simulink model "AcasXuClosedLoop_all_comp"  (Simulink 10.0)
settings: Solver=VariableStepAuto  StartTime=0.0  StopTime=20  RelTol=1e-3
libraries: neural x14040, simulink x2
(part 21 of 534)

== system AcasXuClosedLoop_all_comp/networks/Networks_m_5/AcasXu_1_5/Layer 3 ==
  "a{2} " : Inport  PortDimensions=50
      mask Display: e = 0.06;c1 = [120 230 180]/255;c2 = ([120 230 180]+0)/(256+128);c3 = ([120 230 180]+128)/(256+128);patch([0 1 1 0],[0 0 1 1],c1);patch([0 1 1 1-e 1-e 0+e],[0 0 1 1-e 0+e 0+e],c2);patch([0 0 1 1-e 0+e 0+e],[0 1 1 1-e 1-e 0+e],c3);plot([0 1 1 0 0],[0 0 1 1 0]);
  Delays 1 : Subsystem
      mask Display: e = 0.06;c1 = [120 150 230]/255;c2 = ([120 150 230]+0)/383;c3 = ([120 150 230]+128)/383;patch([0 1 1 0],[0 0 1 1],c1);patch([0 1 1 1-e 1-e 0+e],[0 0 1 1-e 0+e 0+e],c2);patch([0 0 1 1-e 0+e 0+e],[0 1 1 1-e 1-e 0+e],c3);plot([0 1 1 0 0],[0 0 1 1 0]);disp('0');
  "LW{3,2}" : Subsystem
      mask Display: e = 0.06;c1 = [120 150 230]/255;c2 = ([120 150 230]+0)/383;c3 = ([120 150 230]+128)/383;patch([0 1 1 0],[0 0 1 1],c1);patch([0 1 1 1-e 1-e 0+e],[0 0 1 1-e 0+e 0+e],c2);patch([0 0 1 1-e 0+e 0+e],[0 1 1 1-e 1-e 0+e],c3);plot([0 1 1 0 0],[0 0 1 1 0]);disp('W');
  "b{3}" : Constant  Value=[-0.417030999999999985039522698571090586483478546142578125;0.051416999999999997428279385758287389762699604034423828125;-0.1922000000000000097255536957163712941110134124755859375;-0.0247398999999999986976195742727213655598461627960205078125;0.321873000000000020204282691338448785245418548583984375;0.054871999999999997166266751946750446222722530364990234375;0.1382190000000000085211837586030014790594577789306640625;-0.00863861999999999963240071565451216883957386016845703125;0.2219170000000000031459279625778435729444026947021484375;0.27942699999999998095034925427171401679515838623046875;-0.12495699999999999862954069840270676650106906890869140625;-0.401002000000000025092816713367938064038753509521484375;-0.005846829999999999909199299708006947184912860393524169921875;-0.1967190000000000049684700798025005497038364410400390625;-0.11063299999999999523314642146942787803709506988525390625;-0.503530000000000033111291486420668661594390869140625;-0.278268000000000015337064951381762512028217315673828125;-0.326998999999999984122922569440561346709728240966796875;-0.4205360000000000209041672860621474683284759521484375;-0.49979099999999998527044908769312314689159393310546875;-0.0027478500000000000320909965267901498009450733661651611328125;-0.595558000000000031803892852622084319591522216796875;-0.2478019999999999944950701546986238099634647369384765625;-0.0493603999999999987213783469996997155249118804931640625;-0.294870000000000020978774273316957987844944000244140625;0.0196781000000000004523936780742587870918214321136474609375;-0.2268429999999999890913926492430618964135646820068359375;0.323292999999999997040589505559182725846767425537109375;0.1608799999999999952304818862103275023400783538818359375;-0.038599300000000003052491592825390398502349853515625;-0.21826000000000000955679979597334749996662139892578125;0.269465999999999983316456564352847635746002197265625;0.1498879999999999934612304741676780395209789276123046875;-0.332399999999999973265829567026230506598949432373046875;0.2423619999999999941042716500305687077343463897705078125;-0.0757377999999999940161643507963162846863269805908203125;0.1714729999999999865423205847037024796009063720703125;-0.08564599999999999990762944435118697583675384521484375;-0.12559500000000001218580791828571818768978118896484375;-0.018844699999999998951683011227942188270390033721923828125;-0.1395280000000000131255006863284506835043430328369140625;0.291113000000000010647482895365101285278797149658203125;-0.03876670000000000115836229497290332801640033721923828125;0.317319999999999990958343687452725134789943695068359375;0.040440799999999998970423575883614830672740936279296875;-0.407876999999999989565679925362928770482540130615234375;-0.04297800000000000231192842647942597977817058563232421875;0.10669399999999999717470444693390163592994213104248046875;0.1600880000000000080717654782347381114959716796875;0.076302499999999995328181512377341277897357940673828125]
      mask Display: e = 0.06;c1 = [120 150 230]/255;c2 = ([120 150 230]+0)/383;c3 = ([120 150 230]+128)/383;patch([0 1 1 0],[0 0 1 1],c1);patch([0 1 1 1-e 1-e 0+e],[0 0 1 1-e 0+e 0+e],c2);patch([0 0 1 1-e 0+e 0+e],[0 1 1 1-e 1-e 0+e],c3);plot([0 1 1 0 0],[0 0 1 1 0]);disp('b');
  netsum : Sum  Inputs=2  SaturateOnIntegerOverflow=on  InputSameDT=on  OutDataTypeStr=Inherit: Same as first input
      mask Display: e = 0.06;c1 = [120 150 230]/255;c2 = ([120 150 230]+0)/383;c3 = ([120 150 230]+128)/383;patch([0 1 1 0],[0 0 1 1],c1);patch([0 1 1 1-e 1-e 0+e],[0 0 1 1-e 0+e 0+e],c2);patch([0 0 1 1-e 0+e 0+e],[0 1 1 1-e 1-e 0+e],c3);plot([0 1 1 0 0],[0 0 1 1 0]);disp('+');
  poslin : neural/Transfer Functions/poslin
      settings: SourceProductName=Deep Learning Toolbox  SourceProductBaseCode=NN
  "a{3}" : Outport  InitialOutput=[0;0;0;0;0;0;0;0;0;0;0;0;0;0;0;0;0;0;0;0;0;0;0;0;0;0;0;0;0;0;0;0;0;0;0;0;0;0;0;0;0;0;0;0;0;0;0;0;0;0]
      mask Display: e = 0.06;c1 = [120 230 180]/255;c2 = ([120 230 180]+0)/(256+128);c3 = ([120 230 180]+128)/(256+128);patch([0 1 1 0],[0 0 1 1],c1);patch([0 1 1 1-e 1-e 0+e],[0 0 1 1-e 0+e 0+e],c2);patch([0 0 1 1-e 0+e 0+e],[0 1 1 1-e 1-e 0+e],c3);plot([0 1 1 0 0],[0 0 1 1 0]);
  wires:
  poslin/1 -> "a{3}"/1
  netsum/1 -> poslin/1
  "b{3}"/1 -> netsum/2
  "LW{3,2}"/1("lz{3,2}") -> netsum/1
  Delays 1/1("pd{3,2}") -> "LW{3,2}"/1("ad{3,2}")
  "a{2} "/1 -> Delays 1/1("p{3}")

== system AcasXuClosedLoop_all_comp/networks/Networks_m_5/AcasXu_1_5/Layer 4 ==
  "a{3} " : Inport  PortDimensions=50
      mask Display: e = 0.06;c1 = [120 230 180]/255;c2 = ([120 230 180]+0)/(256+128);c3 = ([120 230 180]+128)/(256+128);patch([0 1 1 0],[0 0 1 1],c1);patch([0 1 1 1-e 1-e 0+e],[0 0 1 1-e 0+e 0+e],c2);patch([0 0 1 1-e 0+e 0+e],[0 1 1 1-e 1-e 0+e],c3);plot([0 1 1 0 0],[0 0 1 1 0]);
  Delays 1 : Subsystem
      mask Display: e = 0.06;c1 = [120 150 230]/255;c2 = ([120 150 230]+0)/383;c3 = ([120 150 230]+128)/383;patch([0 1 1 0],[0 0 1 1],c1);patch([0 1 1 1-e 1-e 0+e],[0 0 1 1-e 0+e 0+e],c2);patch([0 0 1 1-e 0+e 0+e],[0 1 1 1-e 1-e 0+e],c3);plot([0 1 1 0 0],[0 0 1 1 0]);disp('0');
  "LW{4,3}" : Subsystem
      mask Display: e = 0.06;c1 = [120 150 230]/255;c2 = ([120 150 230]+0)/383;c3 = ([120 150 230]+128)/383;patch([0 1 1 0],[0 0 1 1],c1);patch([0 1 1 1-e 1-e 0+e],[0 0 1 1-e 0+e 0+e],c2);patch([0 0 1 1-e 0+e 0+e],[0 1 1 1-e 1-e 0+e],c3);plot([0 1 1 0 0],[0 0 1 1 0]);disp('W');
  "b{4}" : Constant  Value=[-0.3807139999999999968594011079403571784496307373046875;0.769236999999999948585127640399150550365447998046875;0.11294200000000000072564176889500231482088565826416015625;-0.298995999999999984009235731718945316970348358154296875;-0.1376940000000000108304476498233270831406116485595703125;-0.2200870000000000048512305284020840190351009368896484375;-0.0667924000000000017696066834105295129120349884033203125;-0.1035719999999999973994135871180333197116851806640625;-0.4381939999999999724167309977929107844829559326171875;-0.197091999999999989423571378210908733308315277099609375;-0.023435299999999999187583199500295449979603290557861328125;-0.0131678999999999997438937526794688892550766468048095703125;0.295812000000000019372947690499131567776203155517578125;0.2067740000000000133439925775746814906597137451171875;-0.825668999999999986272314345114864408969879150390625;-0.1489729999999999943138817570797982625663280487060546875;-0.12124100000000000154631862869791802950203418731689453125;-0.164737999999999995548449760462972335517406463623046875;-0.52807199999999998585309413101640529930591583251953125;0.2460940000000000071889161290528136305510997772216796875;0.11102299999999999668975902977763325907289981842041015625;0.08866369999999999806927775125586776994168758392333984375;0.4474650000000000016342482922482304275035858154296875;0.0499510999999999982801313080926775000989437103271484375;0.477864999999999984225951266125775873661041259765625;-0.1715270000000000127915456005212035961449146270751953125;0.338029999999999997140065488565596751868724822998046875;-0.318321000000000020602186623364104889333248138427734375;-0.302983999999999975560882603531354106962680816650390625;0.07483410000000000061870508716310723684728145599365234375;-0.0716896999999999950947682236801483668386936187744140625;-0.68828999999999995740296299118199385702610015869140625;0.436628999999999989345411677277297712862491607666015625;-0.98602800000000001556799134050379507243633270263671875;-0.00702441000000000002889688488494357443414628505706787109375;-0.67567399999999999682387397115235216915607452392578125;-0.472779000000000004799716180059476755559444427490234375;0.76656299999999999439381781485280953347682952880859375;-0.01495920000000000062156946256664014072157442569732666015625;-0.01252909999999999961228791534040283295325934886932373046875;-0.339034000000000002028599510595086030662059783935546875;0.1361610000000000042508219166847993619740009307861328125;0.30265799999999998259880840123514644801616668701171875;-0.329135000000000010889067425523535348474979400634765625;-0.01919679999999999997495336856445646844804286956787109375;0.10113600000000000367350594387971796095371246337890625;-0.0011569399999999999649469284435099325492046773433685302734375;0.313553000000000026137314534935285337269306182861328125;-1.142490000000000005542233338928781449794769287109375;0.267479999999999995541344333105371333658695220947265625]
      mask Display: e = 0.06;c1 = [120 150 230]/255;c2 = ([120 150 230]+0)/383;c3 = ([120 150 230]+128)/383;patch([0 1 1 0],[0 0 1 1],c1);patch([0 1 1 1-e 1-e 0+e],[0 0 1 1-e 0+e 0+e],c2);patch([0 0 1 1-e 0+e 0+e],[0 1 1 1-e 1-e 0+e],c3);plot([0 1 1 0 0],[0 0 1 1 0]);disp('b');
  netsum : Sum  Inputs=2  SaturateOnIntegerOverflow=on  InputSameDT=on  OutDataTypeStr=Inherit: Same as first input
      mask Display: e = 0.06;c1 = [120 150 230]/255;c2 = ([120 150 230]+0)/383;c3 = ([120 150 230]+128)/383;patch([0 1 1 0],[0 0 1 1],c1);patch([0 1 1 1-e 1-e 0+e],[0 0 1 1-e 0+e 0+e],c2);patch([0 0 1 1-e 0+e 0+e],[0 1 1 1-e 1-e 0+e],c3);plot([0 1 1 0 0],[0 0 1 1 0]);disp('+');
  poslin : neural/Transfer Functions/poslin
      settings: SourceProductName=Deep Learning Toolbox  SourceProductBaseCode=NN
  "a{4}" : Outport  InitialOutput=[0;0;0;0;0;0;0;0;0;0;0;0;0;0;0;0;0;0;0;0;0;0;0;0;0;0;0;0;0;0;0;0;0;0;0;0;0;0;0;0;0;0;0;0;0;0;0;0;0;0]
      mask Display: e = 0.06;c1 = [120 230 180]/255;c2 = ([120 230 180]+0)/(256+128);c3 = ([120 230 180]+128)/(256+128);patch([0 1 1 0],[0 0 1 1],c1);patch([0 1 1 1-e 1-e 0+e],[0 0 1 1-e 0+e 0+e],c2);patch([0 0 1 1-e 0+e 0+e],[0 1 1 1-e 1-e 0+e],c3);plot([0 1 1 0 0],[0 0 1 1 0]);
  wires:
  poslin/1 -> "a{4}"/1
  netsum/1 -> poslin/1
  "b{4}"/1 -> netsum/2
  "LW{4,3}"/1("lz{4,3}") -> netsum/1
  Delays 1/1("pd{4,3}") -> "LW{4,3}"/1("ad{4,3}")
  "a{3} "/1 -> Delays 1/1("p{4}")

== system AcasXuClosedLoop_all_comp/networks/Networks_m_5/AcasXu_1_5/Layer 5 ==
  "a{4} " : Inport  PortDimensions=50
      mask Display: e = 0.06;c1 = [120 230 180]/255;c2 = ([120 230 180]+0)/(256+128);c3 = ([120 230 180]+128)/(256+128);patch([0 1 1 0],[0 0 1 1],c1);patch([0 1 1 1-e 1-e 0+e],[0 0 1 1-e 0+e 0+e],c2);patch([0 0 1 1-e 0+e 0+e],[0 1 1 1-e 1-e 0+e],c3);plot([0 1 1 0 0],[0 0 1 1 0]);
  Delays 1 : Subsystem
      mask Display: e = 0.06;c1 = [120 150 230]/255;c2 = ([120 150 230]+0)/383;c3 = ([120 150 230]+128)/383;patch([0 1 1 0],[0 0 1 1],c1);patch([0 1 1 1-e 1-e 0+e],[0 0 1 1-e 0+e 0+e],c2);patch([0 0 1 1-e 0+e 0+e],[0 1 1 1-e 1-e 0+e],c3);plot([0 1 1 0 0],[0 0 1 1 0]);disp('0');
  "LW{5,4}" : Subsystem
      mask Display: e = 0.06;c1 = [120 150 230]/255;c2 = ([120 150 230]+0)/383;c3 = ([120 150 230]+128)/383;patch([0 1 1 0],[0 0 1 1],c1);patch([0 1 1 1-e 1-e 0+e],[0 0 1 1-e 0+e 0+e],c2);patch([0 0 1 1-e 0+e 0+e],[0 1 1 1-e 1-e 0+e],c3);plot([0 1 1 0 0],[0 0 1 1 0]);disp('W');
  "b{5}" : Constant  Value=[-0.055169999999999996820321257473551668226718902587890625;-0.41405999999999998362198994072969071567058563232421875;0.0014745900000000000153177470707532847882248461246490478515625;0.270907999999999982154719191385083831846714019775390625;0.266349000000000002419398015263141132891178131103515625;0.339114999999999999769073610877967439591884613037109375;-1.0929800000000000625988150204648263752460479736328125;-0.11807499999999999940047956670241546817123889923095703125;0.32457999999999997964295062047312967479228973388671875;0.35002699999999997704236420759116299450397491455078125;-0.435273999999999994248156554021988995373249053955078125;-0.3247189999999999798774297232739627361297607421875;0.1182249999999999967581487680945429019629955291748046875;-0.124670000000000003037570195374428294599056243896484375;-0.011235900000000000054090065759737626649439334869384765625;0.400903000000000009350742402602918446063995361328125;-0.07515719999999999345607903933341731317341327667236328125;0.1054920000000000024353852268177433870732784271240234375;0.1665290000000000103508313031852594576776027679443359375;-1.8045299999999999673860884286114014685153961181640625;0.2429929999999999867821287580227362923324108123779296875;0.9974089999999999900381908446433953940868377685546875;0.1574979999999999991100452234604745171964168548583984375;-0.30226399999999997714184019059757702052593231201171875;-0.313302000000000024915181029427913017570972442626953125;0.2015280000000000126814114764783880673348903656005859375;1.3892700000000000049027448767446912825107574462890625;-0.1532509999999999983355536414819653145968914031982421875;-0.150237000000000009425349389857728965580463409423828125;-0.2088440000000000018598456108520622365176677703857421875;-0.02673419999999999963069541308868792839348316192626953125;0.71494599999999997042010591030702926218509674072265625;0.0299004999999999999948929740867242799140512943267822265625;0.08596589999999999787139159934667986817657947540283203125;0.179721999999999992869703646647394634783267974853515625;0.05434239999999999903845804283264442346990108489990234375;0.6289379999999999970583530739531852304935455322265625;0.355820000000000025153212845907546579837799072265625;0.042970500000000001750155576019096770323812961578369140625;-0.1282999999999999973798736618846305646002292633056640625;-0.49241699999999999359800995080149732530117034912109375;0.7115080000000000293169932774617336690425872802734375;-0.09608129999999999448778709165708278305828571319580078125;0.354057000000000010597744903861894272267818450927734375;0.2978899999999999881339363128063268959522247314453125;-0.055887399999999996802468871237579151056706905364990234375;0.64739500000000005375255796025157906115055084228515625;0.09175229999999999497273250881335115991532802581787109375;-0.1348080000000000111537445945941726677119731903076171875;-0.457637000000000015997869695638655684888362884521484375]
      mask Display: e = 0.06;c1 = [120 150 230]/255;c2 = ([120 150 230]+0)/383;c3 = ([120 150 230]+128)/383;patch([0 1 1 0],[0 0 1 1],c1);patch([0 1 1 1-e 1-e 0+e],[0 0 1 1-e 0+e 0+e],c2);patch([0 0 1 1-e 0+e 0+e],[0 1 1 1-e 1-e 0+e],c3);plot([0 1 1 0 0],[0 0 1 1 0]);disp('b');
  netsum : Sum  Inputs=2  SaturateOnIntegerOverflow=on  InputSameDT=on  OutDataTypeStr=Inherit: Same as first input
      mask Display: e = 0.06;c1 = [120 150 230]/255;c2 = ([120 150 230]+0)/383;c3 = ([120 150 230]+128)/383;patch([0 1 1 0],[0 0 1 1],c1);patch([0 1 1 1-e 1-e 0+e],[0 0 1 1-e 0+e 0+e],c2);patch([0 0 1 1-e 0+e 0+e],[0 1 1 1-e 1-e 0+e],c3);plot([0 1 1 0 0],[0 0 1 1 0]);disp('+');
  poslin : neural/Transfer Functions/poslin
      settings: SourceProductName=Deep Learning Toolbox  SourceProductBaseCode=NN
  "a{5}" : Outport  InitialOutput=[0;0;0;0;0;0;0;0;0;0;0;0;0;0;0;0;0;0;0;0;0;0;0;0;0;0;0;0;0;0;0;0;0;0;0;0;0;0;0;0;0;0;0;0;0;0;0;0;0;0]
      mask Display: e = 0.06;c1 = [120 230 180]/255;c2 = ([120 230 180]+0)/(256+128);c3 = ([120 230 180]+128)/(256+128);patch([0 1 1 0],[0 0 1 1],c1);patch([0 1 1 1-e 1-e 0+e],[0 0 1 1-e 0+e 0+e],c2);patch([0 0 1 1-e 0+e 0+e],[0 1 1 1-e 1-e 0+e],c3);plot([0 1 1 0 0],[0 0 1 1 0]);
  wires:
  poslin/1 -> "a{5}"/1
  netsum/1 -> poslin/1
  "b{5}"/1 -> netsum/2
  "LW{5,4}"/1("lz{5,4}") -> netsum/1
  Delays 1/1("pd{5,4}") -> "LW{5,4}"/1("ad{5,4}")
  "a{4} "/1 -> Delays 1/1("p{5}")

== system AcasXuClosedLoop_all_comp/networks/Networks_m_5/AcasXu_1_5/Layer 6 ==
  "a{5} " : Inport  PortDimensions=50
      mask Display: e = 0.06;c1 = [120 230 180]/255;c2 = ([120 230 180]+0)/(256+128);c3 = ([120 230 180]+128)/(256+128);patch([0 1 1 0],[0 0 1 1],c1);patch([0 1 1 1-e 1-e 0+e],[0 0 1 1-e 0+e 0+e],c2);patch([0 0 1 1-e 0+e 0+e],[0 1 1 1-e 1-e 0+e],c3);plot([0 1 1 0 0],[0 0 1 1 0]);
  Delays 1 : Subsystem
      mask Display: e = 0.06;c1 = [120 150 230]/255;c2 = ([120 150 230]+0)/383;c3 = ([120 150 230]+128)/383;patch([0 1 1 0],[0 0 1 1],c1);patch([0 1 1 1-e 1-e 0+e],[0 0 1 1-e 0+e 0+e],c2);patch([0 0 1 1-e 0+e 0+e],[0 1 1 1-e 1-e 0+e],c3);plot([0 1 1 0 0],[0 0 1 1 0]);disp('0');
  "LW{6,5}" : Subsystem
      mask Display: e = 0.06;c1 = [120 150 230]/255;c2 = ([120 150 230]+0)/383;c3 = ([120 150 230]+128)/383;patch([0 1 1 0],[0 0 1 1],c1);patch([0 1 1 1-e 1-e 0+e],[0 0 1 1-e 0+e 0+e],c2);patch([0 0 1 1-e 0+e 0+e],[0 1 1 1-e 1-e 0+e],c3);plot([0 1 1 0 0],[0 0 1 1 0]);disp('W');
  "b{6}" : Constant  Value=[-0.212903000000000008906653192752855829894542694091796875;0.1829119999999999912621007069901679642498493194580078125;0.29444700000000001427480356142041273415088653564453125;0.42106100000000001859490339484182186424732208251953125;-0.05925729999999999886295398709989967755973339080810546875;-0.96771399999999996310862115933559834957122802734375;-0.017947999999999998677058243856663466431200504302978515625;0.51803999999999994496846511538024060428142547607421875;0.0897030000000000049542592250872985459864139556884765625;0.061536000000000000420552481728009297512471675872802734375;0.311950000000000005062616992290713824331760406494140625;-0.413208999999999992969179629653808660805225372314453125;0.3383249999999999868549593884381465613842010498046875;-0.486316000000000026037838551928871311247348785400390625;0.2037269999999999914308546067331917583942413330078125;-0.0403260000000000007336353746723034419119358062744140625;-0.307506000000000001559641304993419907987117767333984375;0.06562410000000000465103511260167579166591167449951171875;-0.034883900000000002405986521125669241882860660552978515625;0.34183099999999999596411726088263094425201416015625;-0.0035042400000000000458155735572063349536620080471038818359375;0.0245180000000000015980550216454503242857754230499267578125;0.661869999999999958362195684458129107952117919921875;-0.623188999999999992951416061259806156158447265625;-0.11044900000000000550581802372107631526887416839599609375;-0.057677899999999997227018155854239012114703655242919921875;-0.03260649999999999659738847412882023490965366363525390625;-0.1454660000000000119602105996818863786756992340087890625;-0.32797799999999999176480969254043884575366973876953125;0.204077000000000008395062423005583696067333221435546875;-0.021273699999999999554756158204327221028506755828857421875;0.382120000000000015205614545266143977642059326171875;-0.1458250000000000101696429055664339102804660797119140625;-0.0122827999999999999680699858117804978974163532257080078125;-0.51167799999999996618527120517683215439319610595703125;-0.2363280000000000102833297432880499400198459625244140625;-0.0236589000000000000689670542897147242911159992218017578125;-0.57806900000000005501732402990455739200115203857421875;-0.05386180000000000134274813490264932624995708465576171875;1.28347999999999995424104781704954802989959716796875;-0.238109999999999988329335565140354447066783905029296875;-0.02524300000000000154809498553731827996671199798583984375;-0.16046199999999999352695567722548730671405792236328125;-0.01005679999999999942039696776419077650643885135650634765625;-0.32135999999999997900346215828903950750827789306640625;-0.94411999999999995925037410415825434029102325439453125;-0.569733999999999962682295517879538238048553466796875;-0.0232010999999999988074872447896268568001687526702880859375;0.5037599999999999855759824640699662268161773681640625;0.430217000000000016068923969214665703475475311279296875]
      mask Display: e = 0.06;c1 = [120 150 230]/255;c2 = ([120 150 230]+0)/383;c3 = ([120 150 230]+128)/383;patch([0 1 1 0],[0 0 1 1],c1);patch([0 1 1 1-e 1-e 0+e],[0 0 1 1-e 0+e 0+e],c2);patch([0 0 1 1-e 0+e 0+e],[0 1 1 1-e 1-e 0+e],c3);plot([0 1 1 0 0],[0 0 1 1 0]);disp('b');
  netsum : Sum  Inputs=2  SaturateOnIntegerOverflow=on  InputSameDT=on  OutDataTypeStr=Inherit: Same as first input
      mask Display: e = 0.06;c1 = [120 150 230]/255;c2 = ([120 150 230]+0)/383;c3 = ([120 150 230]+128)/383;patch([0 1 1 0],[0 0 1 1],c1);patch([0 1 1 1-e 1-e 0+e],[0 0 1 1-e 0+e 0+e],c2);patch([0 0 1 1-e 0+e 0+e],[0 1 1 1-e 1-e 0+e],c3);plot([0 1 1 0 0],[0 0 1 1 0]);disp('+');
  poslin : neural/Transfer Functions/poslin
      settings: SourceProductName=Deep Learning Toolbox  SourceProductBaseCode=NN
  "a{6}" : Outport  InitialOutput=[0;0;0;0;0;0;0;0;0;0;0;0;0;0;0;0;0;0;0;0;0;0;0;0;0;0;0;0;0;0;0;0;0;0;0;0;0;0;0;0;0;0;0;0;0;0;0;0;0;0]
      mask Display: e = 0.06;c1 = [120 230 180]/255;c2 = ([120 230 180]+0)/(256+128);c3 = ([120 230 180]+128)/(256+128);patch([0 1 1 0],[0 0 1 1],c1);patch([0 1 1 1-e 1-e 0+e],[0 0 1 1-e 0+e 0+e],c2);patch([0 0 1 1-e 0+e 0+e],[0 1 1 1-e 1-e 0+e],c3);plot([0 1 1 0 0],[0 0 1 1 0]);
  wires:
  poslin/1 -> "a{6}"/1
  netsum/1 -> poslin/1
  "b{6}"/1 -> netsum/2
  "LW{6,5}"/1("lz{6,5}") -> netsum/1
  Delays 1/1("pd{6,5}") -> "LW{6,5}"/1("ad{6,5}")
  "a{5} "/1 -> Delays 1/1("p{6}")

== system AcasXuClosedLoop_all_comp/networks/Networks_m_5/AcasXu_1_5/Layer 7 ==
  "a{6} " : Inport  PortDimensions=50
      mask Display: e = 0.06;c1 = [120 230 180]/255;c2 = ([120 230 180]+0)/(256+128);c3 = ([120 230 180]+128)/(256+128);patch([0 1 1 0],[0 0 1 1],c1);patch([0 1 1 1-e 1-e 0+e],[0 0 1 1-e 0+e 0+e],c2);patch([0 0 1 1-e 0+e 0+e],[0 1 1 1-e 1-e 0+e],c3);plot([0 1 1 0 0],[0 0 1 1 0]);
  Delays 1 : Subsystem
      mask Display: e = 0.06;c1 = [120 150 230]/255;c2 = ([120 150 230]+0)/383;c3 = ([120 150 230]+128)/383;patch([0 1 1 0],[0 0 1 1],c1);patch([0 1 1 1-e 1-e 0+e],[0 0 1 1-e 0+e 0+e],c2);patch([0 0 1 1-e 0+e 0+e],[0 1 1 1-e 1-e 0+e],c3);plot([0 1 1 0 0],[0 0 1 1 0]);disp('0');
  "LW{7,6}" : Subsystem
      mask Display: e = 0.06;c1 = [120 150 230]/255;c2 = ([120 150 230]+0)/383;c3 = ([120 150 230]+128)/383;patch([0 1 1 0],[0 0 1 1],c1);patch([0 1 1 1-e 1-e 0+e],[0 0 1 1-e 0+e 0+e],c2);patch([0 0 1 1-e 0+e 0+e],[0 1 1 1-e 1-e 0+e],c3);plot([0 1 1 0 0],[0 0 1 1 0]);disp('W');
  "b{7}" : Constant  Value=[-0.01177249999999999997835065101980944746173918247222900390625;-0.0173447000000000010888623336313685285858809947967529296875;-0.016608299999999999452260368570932769216597080230712890625;-0.016006499999999999894750857265535159967839717864990234375;-0.0142433999999999998109512233668283442966639995574951171875]
      mask Display: e = 0.06;c1 = [120 150 230]/255;c2 = ([120 150 230]+0)/383;c3 = ([120 150 230]+128)/383;patch([0 1 1 0],[0 0 1 1],c1);patch([0 1 1 1-e 1-e 0+e],[0 0 1 1-e 0+e 0+e],c2);patch([0 0 1 1-e 0+e 0+e],[0 1 1 1-e 1-e 0+e],c3);plot([0 1 1 0 0],[0 0 1 1 0]);disp('b');
  netsum : Sum  Inputs=2  SaturateOnIntegerOverflow=on  InputSameDT=on  OutDataTypeStr=Inherit: Same as first input
      mask Display: e = 0.06;c1 = [120 150 230]/255;c2 = ([120 150 230]+0)/383;c3 = ([120 150 230]+128)/383;patch([0 1 1 0],[0 0 1 1],c1);patch([0 1 1 1-e 1-e 0+e],[0 0 1 1-e 0+e 0+e],c2);patch([0 0 1 1-e 0+e 0+e],[0 1 1 1-e 1-e 0+e],c3);plot([0 1 1 0 0],[0 0 1 1 0]);disp('+');
  purelin : neural/Transfer Functions/purelin
      settings: SourceProductName=Deep Learning Toolbox
  "a{7}" : Outport  InitialOutput=[0;0;0;0;0]
      mask Display: e = 0.06;c1 = [120 230 180]/255;c2 = ([120 230 180]+0)/(256+128);c3 = ([120 230 180]+128)/(256+128);patch([0 1 1 0],[0 0 1 1],c1);patch([0 1 1 1-e 1-e 0+e],[0 0 1 1-e 0+e 0+e],c2);patch([0 0 1 1-e 0+e 0+e],[0 1 1 1-e 1-e 0+e],c3);plot([0 1 1 0 0],[0 0 1 1 0]);
  wires:
  purelin/1 -> "a{7}"/1
  netsum/1 -> purelin/1
  "b{7}"/1 -> netsum/2
  "LW{7,6}"/1("lz{7,6}") -> netsum/1
  Delays 1/1("pd{7,6}") -> "LW{7,6}"/1("ad{7,6}")
  "a{6} "/1 -> Delays 1/1("p{7}")

== system AcasXuClosedLoop_all_comp/networks/Networks_m_5/AcasXu_1_5/Process Input 1 ==
  x : Inport  PortDimensions=5
      mask Display: e = 0.06;c1 = [120 230 180]/255;c2 = ([120 230 180]+0)/(256+128);c3 = ([120 230 180]+128)/(256+128);patch([0 1 1 0],[0 0 1 1],c1);patch([0 1 1 1-e 1-e 0+e],[0 0 1 1-e 0+e 0+e],c2);patch([0 0 1 1-e 0+e 0+e],[0 1 1 1-e 1-e 0+e],c3);plot([0 1 1 0 0],[0 0 1 1 0]);
  p : Outport  PortDimensions=5
      mask Display: e = 0.06;c1 = [120 230 180]/255;c2 = ([120 230 180]+0)/(256+128);c3 = ([120 230 180]+128)/(256+128);patch([0 1 1 0],[0 0 1 1],c1);patch([0 1 1 1-e 1-e 0+e],[0 0 1 1-e 0+e 0+e],c2);patch([0 0 1 1-e 0+e 0+e],[0 1 1 1-e 1-e 0+e],c3);plot([0 1 1 0 0],[0 0 1 1 0]);
  wires:
  x/1 -> p/1

== system AcasXuClosedLoop_all_comp/networks/Networks_m_5/AcasXu_1_5/Process Output 1 ==
  a : Inport  PortDimensions=5
      mask Display: e = 0.06;c1 = [120 230 180]/255;c2 = ([120 230 180]+0)/(256+128);c3 = ([120 230 180]+128)/(256+128);patch([0 1 1 0],[0 0 1 1],c1);patch([0 1 1 1-e 1-e 0+e],[0 0 1 1-e 0+e 0+e],c2);patch([0 0 1 1-e 0+e 0+e],[0 1 1 1-e 1-e 0+e],c3);plot([0 1 1 0 0],[0 0 1 1 0]);
  y : Outport  PortDimensions=5
      mask Display: e = 0.06;c1 = [120 230 180]/255;c2 = ([120 230 180]+0)/(256+128);c3 = ([120 230 180]+128)/(256+128);patch([0 1 1 0],[0 0 1 1],c1);patch([0 1 1 1-e 1-e 0+e],[0 0 1 1-e 0+e 0+e],c2);patch([0 0 1 1-e 0+e 0+e],[0 1 1 1-e 1-e 0+e],c3);plot([0 1 1 0 0],[0 0 1 1 0]);
  wires:
  a/1 -> y/1

== system AcasXuClosedLoop_all_comp/networks/Networks_m_5/AcasXu_2_5/Layer 1 ==
  "p{1}" : Inport  PortDimensions=5
      mask Display: e = 0.06;c1 = [120 230 180]/255;c2 = ([120 230 180]+0)/(256+128);c3 = ([120 230 180]+128)/(256+128);patch([0 1 1 0],[0 0 1 1],c1);patch([0 1 1 1-e 1-e 0+e],[0 0 1 1-e 0+e 0+e],c2);patch([0 0 1 1-e 0+e 0+e],[0 1 1 1-e 1-e 0+e],c3);plot([0 1 1 0 0],[0 0 1 1 0]);
  Delays 1 : Subsystem
      mask Display: e = 0.06;c1 = [120 150 230]/255;c2 = ([120 150 230]+0)/383;c3 = ([120 150 230]+128)/383;patch([0 1 1 0],[0 0 1 1],c1);patch([0 1 1 1-e 1-e 0+e],[0 0 1 1-e 0+e 0+e],c2);patch([0 0 1 1-e 0+e 0+e],[0 1 1 1-e 1-e 0+e],c3);plot([0 1 1 0 0],[0 0 1 1 0]);disp('0');
  "IW{1,1}" : Subsystem
      mask Display: e = 0.06;c1 = [120 150 230]/255;c2 = ([120 150 230]+0)/383;c3 = ([120 150 230]+128)/383;patch([0 1 1 0],[0 0 1 1],c1);patch([0 1 1 1-e 1-e 0+e],[0 0 1 1-e 0+e 0+e],c2);patch([0 0 1 1-e 0+e 0+e],[0 1 1 1-e 1-e 0+e],c3);plot([0 1 1 0 0],[0 0 1 1 0]);disp('W');
  "b{1}" : Constant  Value=[-0.0934323999999999987853271932181087322533130645751953125;-0.68851399999999995937827179659507237374782562255859375;0.181541000000000007918998790046316571533679962158203125;-0.17247000000000001218580791828571818768978118896484375;-0.1560479999999999922710713917695102281868457794189453125;0.12035099999999999964561681053965003229677677154541015625;0.06389630000000000309423597855129628442227840423583984375;-0.086717199999999994286525861753034405410289764404296875;0.226731999999999989103827147118863649666309356689453125;-0.271637999999999990574650610142271034419536590576171875;0.1380530000000000090398799557078746147453784942626953125;-0.0289674000000000007870593066172659746371209621429443359375;-0.052192099999999998216626551084118545986711978912353515625;0.0257320000000000013995471448424723348580300807952880859375;-0.073908899999999999597122268824023194611072540283203125;-0.254815999999999986957988085123361088335514068603515625;-0.29719899999999999096189640113152563571929931640625;-0.37860199999999999409538986583356745541095733642578125;0.275386999999999992905230783435399644076824188232421875;0.07508850000000000246558329308754764497280120849609375;0.2205519999999999980477838334991247393190860748291015625;0.08967540000000000233182362308070878498256206512451171875;0.11587699999999999389554972140103927813470363616943359375;-0.68539600000000000523669996255193836987018585205078125;0.007177379999999999697635200135437116841785609722137451171875;0.119696999999999997843502796968095935881137847900390625;0.0220079999999999997850608224325696937739849090576171875;0.48674600000000001198685595227289013564586639404296875;0.0803882999999999958706808911301777698099613189697265625;-0.00837524000000000080901063626015456975437700748443603515625;0.051856699999999998629274244876796728931367397308349609375;0.007693120000000000340001360399355689878575503826141357421875;-0.1534590000000000120650156532065011560916900634765625;-0.143386999999999986687981845534523017704486846923828125;-0.07607619999999999660378335875066113658249378204345703125;-0.055888000000000000178079773149875109083950519561767578125;-0.000299668000000000004680311693761041169636882841587066650390625;0.1378749999999999975575093458246556110680103302001953125;-0.06373510000000000286224377532562357373535633087158203125;0.09505560000000000397957222730838111601769924163818359375;0.2410200000000000120081722343456931412220001220703125;-0.1569319999999999881712398064337321557104587554931640625;-0.258384000000000002561506562415161170065402984619140625;0.503395999999999954610530039644800126552581787109375;-0.278918999999999972505548839762923307716846466064453125;0.02316100000000000103117514527184539474546909332275390625;-0.54474699999999998123456634857575409114360809326171875;0.09261429999999999662652072629498434253036975860595703125;0.17898300000000000320454773827805183827877044677734375;-0.44224099999999999521804738833452574908733367919921875]
      mask Display: e = 0.06;c1 = [120 150 230]/255;c2 = ([120 150 230]+0)/383;c3 = ([120 150 230]+128)/383;patch([0 1 1 0],[0 0 1 1],c1);patch([0 1 1 1-e 1-e 0+e],[0 0 1 1-e 0+e 0+e],c2);patch([0 0 1 1-e 0+e 0+e],[0 1 1 1-e 1-e 0+e],c3);plot([0 1 1 0 0],[0 0 1 1 0]);disp('b');
  netsum : Sum  Inputs=2  SaturateOnIntegerOverflow=on  InputSameDT=on  OutDataTypeStr=Inherit: Same as first input
      mask Display: e = 0.06;c1 = [120 150 230]/255;c2 = ([120 150 230]+0)/383;c3 = ([120 150 230]+128)/383;patch([0 1 1 0],[0 0 1 1],c1);patch([0 1 1 1-e 1-e 0+e],[0 0 1 1-e 0+e 0+e],c2);patch([0 0 1 1-e 0+e 0+e],[0 1 1 1-e 1-e 0+e],c3);plot([0 1 1 0 0],[0 0 1 1 0]);disp('+');
  poslin : neural/Transfer Functions/poslin
      settings: SourceProductName=Deep Learning Toolbox  SourceProductBaseCode=NN
  "a{1}" : Outport  InitialOutput=[0;0;0;0;0;0;0;0;0;0;0;0;0;0;0;0;0;0;0;0;0;0;0;0;0;0;0;0;0;0;0;0;0;0;0;0;0;0;0;0;0;0;0;0;0;0;0;0;0;0]
      mask Display: e = 0.06;c1 = [120 230 180]/255;c2 = ([120 230 180]+0)/(256+128);c3 = ([120 230 180]+128)/(256+128);patch([0 1 1 0],[0 0 1 1],c1);patch([0 1 1 1-e 1-e 0+e],[0 0 1 1-e 0+e 0+e],c2);patch([0 0 1 1-e 0+e 0+e],[0 1 1 1-e 1-e 0+e],c3);plot([0 1 1 0 0],[0 0 1 1 0]);
  wires:
  poslin/1 -> "a{1}"/1
  netsum/1 -> poslin/1
  "b{1}"/1 -> netsum/2
  "IW{1,1}"/1("iz{1,1}") -> netsum/1
  Delays 1/1("pd{1,1}") -> "IW{1,1}"/1("pd{1,1}")
  "p{1}"/1 -> Delays 1/1("p{1}")

== system AcasXuClosedLoop_all_comp/networks/Networks_m_5/AcasXu_2_5/Layer 2 ==
  "a{1} " : Inport  PortDimensions=50
      mask Display: e = 0.06;c1 = [120 230 180]/255;c2 = ([120 230 180]+0)/(256+128);c3 = ([120 230 180]+128)/(256+128);patch([0 1 1 0],[0 0 1 1],c1);patch([0 1 1 1-e 1-e 0+e],[0 0 1 1-e 0+e 0+e],c2);patch([0 0 1 1-e 0+e 0+e],[0 1 1 1-e 1-e 0+e],c3);plot([0 1 1 0 0],[0 0 1 1 0]);
  Delays 1 : Subsystem
      mask Display: e = 0.06;c1 = [120 150 230]/255;c2 = ([120 150 230]+0)/383;c3 = ([120 150 230]+128)/383;patch([0 1 1 0],[0 0 1 1],c1);patch([0 1 1 1-e 1-e 0+e],[0 0 1 1-e 0+e 0+e],c2);patch([0 0 1 1-e 0+e 0+e],[0 1 1 1-e 1-e 0+e],c3);plot([0 1 1 0 0],[0 0 1 1 0]);disp('0');
  "LW{2,1}" : Subsystem
      mask Display: e = 0.06;c1 = [120 150 230]/255;c2 = ([120 150 230]+0)/383;c3 = ([120 150 230]+128)/383;patch([0 1 1 0],[0 0 1 1],c1);patch([0 1 1 1-e 1-e 0+e],[0 0 1 1-e 0+e 0+e],c2);patch([0 0 1 1-e 0+e 0+e],[0 1 1 1-e 1-e 0+e],c3);plot([0 1 1 0 0],[0 0 1 1 0]);disp('W');
  "b{2}" : Constant  Value=[0.77493999999999996219912645756267011165618896484375;-1.035860000000000002984279490192420780658721923828125;-0.6659129999999999771631564726703800261020660400390625;-0.401067000000000006831868404333363287150859832763671875;-0.3490130000000000176640924109960906207561492919921875;-0.06292230000000000045279335836312384344637393951416015625;0.0043870899999999997398436590856363181956112384796142578125;-0.63266699999999997938715523559949360787868499755859375;0.816235999999999961573848850093781948089599609375;-0.2518569999999999975415221342700533568859100341796875;-1.0546599999999999308641918105422519147396087646484375;-0.0090162899999999997213695479558737133629620075225830078125;-0.2937119999999999730988520241226069629192352294921875;-0.0567676000000000013923084907219163142144680023193359375;-0.26603399999999999270272610374377109110355377197265625;-0.11877999999999999669597627871553413569927215576171875;-0.38886500000000001620037437533028423786163330078125;-0.057355499999999996763033749402893590740859508514404296875;-0.37688500000000002554401135057560168206691741943359375;-0.1763800000000000089972473915622686035931110382080078125;-0.315118999999999982453147140404325909912586212158203125;-0.01377920000000000012863043963307063677348196506500244140625;0.11324900000000000244160247575564426369965076446533203125;0.5274739999999999984225951266125775873661041259765625;-0.00671154000000000021397550398205567034892737865447998046875;0.0951660000000000005915268275202834047377109527587890625;0.36432399999999998119193378443014807999134063720703125;1.09447999999999989739762895624153316020965576171875;-0.01527249999999999961752816801663357182405889034271240234375;-0.701780999999999988148147167521528899669647216796875;-0.015941799999999999026822905534572782926261425018310546875;-0.63614499999999996049382389173842966556549072265625;-0.424455999999999999960920149533194489777088165283203125;0.806046999999999957964291752432473003864288330078125;-1.07462999999999997413624441833235323429107666015625;-0.51002800000000003688427341330680064857006072998046875;0.838670000000000026574298317427746951580047607421875;-1.23582999999999998408384271897375583648681640625;-1.082300000000000039790393202565610408782958984375;-1.0480199999999999516120396947371773421764373779296875;-0.2257760000000000044639847374128294177353382110595703125;-0.57211299999999998266986267481115646660327911376953125;-0.53944000000000003058886477447231300175189971923828125;0.9423369999999999802042793817236088216304779052734375;-0.3460929999999999839843667359673418104648590087890625;-0.63220200000000004170175316176027990877628326416015625;-0.2412209999999999909814363263649283908307552337646484375;0.7980840000000000156177293320070020854473114013671875;0.282801000000000024581225943620665930211544036865234375;-0.0454900000000000026556534749033744446933269500732421875]
      mask Display: e = 0.06;c1 = [120 150 230]/255;c2 = ([120 150 230]+0)/383;c3 = ([120 150 230]+128)/383;patch([0 1 1 0],[0 0 1 1],c1);patch([0 1 1 1-e 1-e 0+e],[0 0 1 1-e 0+e 0+e],c2);patch([0 0 1 1-e 0+e 0+e],[0 1 1 1-e 1-e 0+e],c3);plot([0 1 1 0 0],[0 0 1 1 0]);disp('b');
  netsum : Sum  Inputs=2  SaturateOnIntegerOverflow=on  InputSameDT=on  OutDataTypeStr=Inherit: Same as first input
      mask Display: e = 0.06;c1 = [120 150 230]/255;c2 = ([120 150 230]+0)/383;c3 = ([120 150 230]+128)/383;patch([0 1 1 0],[0 0 1 1],c1);patch([0 1 1 1-e 1-e 0+e],[0 0 1 1-e 0+e 0+e],c2);patch([0 0 1 1-e 0+e 0+e],[0 1 1 1-e 1-e 0+e],c3);plot([0 1 1 0 0],[0 0 1 1 0]);disp('+');
  poslin : neural/Transfer Functions/poslin
      settings: SourceProductName=Deep Learning Toolbox  SourceProductBaseCode=NN
  "a{2}" : Outport  InitialOutput=[0;0;0;0;0;0;0;0;0;0;0;0;0;0;0;0;0;0;0;0;0;0;0;0;0;0;0;0;0;0;0;0;0;0;0;0;0;0;0;0;0;0;0;0;0;0;0;0;0;0]
      mask Display: e = 0.06;c1 = [120 230 180]/255;c2 = ([120 230 180]+0)/(256+128);c3 = ([120 230 180]+128)/(256+128);patch([0 1 1 0],[0 0 1 1],c1);patch([0 1 1 1-e 1-e 0+e],[0 0 1 1-e 0+e 0+e],c2);patch([0 0 1 1-e 0+e 0+e],[0 1 1 1-e 1-e 0+e],c3);plot([0 1 1 0 0],[0 0 1 1 0]);
  wires:
  poslin/1 -> "a{2}"/1
  netsum/1 -> poslin/1
  "b{2}"/1 -> netsum/2
  "LW{2,1}"/1("lz{2,1}") -> netsum/1
  Delays 1/1("pd{2,1}") -> "LW{2,1}"/1("ad{2,1}")
  "a{1} "/1 -> Delays 1/1("p{2}")

== system AcasXuClosedLoop_all_comp/networks/Networks_m_5/AcasXu_2_5/Layer 3 ==
  "a{2} " : Inport  PortDimensions=50
      mask Display: e = 0.06;c1 = [120 230 180]/255;c2 = ([120 230 180]+0)/(256+128);c3 = ([120 230 180]+128)/(256+128);patch([0 1 1 0],[0 0 1 1],c1);patch([0 1 1 1-e 1-e 0+e],[0 0 1 1-e 0+e 0+e],c2);patch([0 0 1 1-e 0+e 0+e],[0 1 1 1-e 1-e 0+e],c3);plot([0 1 1 0 0],[0 0 1 1 0]);
  Delays 1 : Subsystem
      mask Display: e = 0.06;c1 = [120 150 230]/255;c2 = ([120 150 230]+0)/383;c3 = ([120 150 230]+128)/383;patch([0 1 1 0],[0 0 1 1],c1);patch([0 1 1 1-e 1-e 0+e],[0 0 1 1-e 0+e 0+e],c2);patch([0 0 1 1-e 0+e 0+e],[0 1 1 1-e 1-e 0+e],c3);plot([0 1 1 0 0],[0 0 1 1 0]);disp('0');
  "LW{3,2}" : Subsystem
      mask Display: e = 0.06;c1 = [120 150 230]/255;c2 = ([120 150 230]+0)/383;c3 = ([120 150 230]+128)/383;patch([0 1 1 0],[0 0 1 1],c1);patch([0 1 1 1-e 1-e 0+e],[0 0 1 1-e 0+e 0+e],c2);patch([0 0 1 1-e 0+e 0+e],[0 1 1 1-e 1-e 0+e],c3);plot([0 1 1 0 0],[0 0 1 1 0]);disp('W');
  "b{3}" : Constant  Value=[0.017563399999999999845368137130208197049796581268310546875;-0.035661699999999997567901033335147076286375522613525390625;-0.674603999999999981440623741946183145046234130859375;0.718156000000000016569856597925536334514617919921875;0.328438999999999980961007395308115519583225250244140625;-0.60492800000000002125233322658459655940532684326171875;0.5249340000000000117097442853264510631561279296875;-0.68299699999999996524735479397349990904331207275390625;-1.1371500000000001051603248924948275089263916015625;-2.427230000000000220694573727087117731571197509765625;-1.359339999999999992752464095246978104114532470703125;0.28417700000000001292477236347622238099575042724609375;-0.28000700000000000589039927945123054087162017822265625;-1.7350399999999999156585772652761079370975494384765625;0.181308999999999997942978779974509961903095245361328125;2.673010000000000108144604382687248289585113525390625;-0.438157999999999991924681808086461387574672698974609375;0.3008100000000000218136619878350757062435150146484375;0.48294599999999998640731746490928344428539276123046875;-0.30930000000000001936228954946273006498813629150390625;-0.911882999999999999118927007657475769519805908203125;-1.536070000000000046469494918710552155971527099609375;0.340861999999999998323119143606163561344146728515625;1.266929999999999889581658862880431115627288818359375;0.254830999999999974203745978229562751948833465576171875;0.1867109999999999880859746781425201334059238433837890625;0.06298020000000000007123190925995004363358020782470703125;-0.69229499999999999371169678852311335504055023193359375;-2.125599999999999933919525574310682713985443115234375;-0.7906109999999999526920646530925296247005462646484375;1.6257200000000000539301936441916041076183319091796875;0.9691229999999999566995256827794946730136871337890625;-0.65317700000000000759570184527547098696231842041015625;-1.1834000000000000074606987254810519516468048095703125;-1.3096399999999999153033058973960578441619873046875;-0.65699600000000002442135382807464338839054107666015625;0.9660860000000000002984279490192420780658721923828125;0.269772999999999985032417271213489584624767303466796875;-0.465776000000000023337776156040490604937076568603515625;-1.365769999999999928519400782533921301364898681640625;-1.923999999999999932498440102790482342243194580078125;-0.9410920000000000396056520912679843604564666748046875;0.505411000000000054654947234666906297206878662109375;-1.5723100000000000964206492426455952227115631103515625;-0.45776600000000000623145979261607863008975982666015625;-1.4048599999999999976552089719916693866252899169921875;-0.32419100000000000694200252837617881596088409423828125;-0.68269699999999999828759200681815855205059051513671875;-0.0218660000000000000197619698383277864195406436920166015625;1.248510000000000008668621376273222267627716064453125]
      mask Display: e = 0.06;c1 = [120 150 230]/255;c2 = ([120 150 230]+0)/383;c3 = ([120 150 230]+128)/383;patch([0 1 1 0],[0 0 1 1],c1);patch([0 1 1 1-e 1-e 0+e],[0 0 1 1-e 0+e 0+e],c2);patch([0 0 1 1-e 0+e 0+e],[0 1 1 1-e 1-e 0+e],c3);plot([0 1 1 0 0],[0 0 1 1 0]);disp('b');
  netsum : Sum  Inputs=2  SaturateOnIntegerOverflow=on  InputSameDT=on  OutDataTypeStr=Inherit: Same as first input
      mask Display: e = 0.06;c1 = [120 150 230]/255;c2 = ([120 150 230]+0)/383;c3 = ([120 150 230]+128)/383;patch([0 1 1 0],[0 0 1 1],c1);patch([0 1 1 1-e 1-e 0+e],[0 0 1 1-e 0+e 0+e],c2);patch([0 0 1 1-e 0+e 0+e],[0 1 1 1-e 1-e 0+e],c3);plot([0 1 1 0 0],[0 0 1 1 0]);disp('+');
  poslin : neural/Transfer Functions/poslin
      settings: SourceProductName=Deep Learning Toolbox  SourceProductBaseCode=NN
  "a{3}" : Outport  InitialOutput=[0;0;0;0;0;0;0;0;0;0;0;0;0;0;0;0;0;0;0;0;0;0;0;0;0;0;0;0;0;0;0;0;0;0;0;0;0;0;0;0;0;0;0;0;0;0;0;0;0;0]
      mask Display: e = 0.06;c1 = [120 230 180]/255;c2 = ([120 230 180]+0)/(256+128);c3 = ([120 230 180]+128)/(256+128);patch([0 1 1 0],[0 0 1 1],c1);patch([0 1 1 1-e 1-e 0+e],[0 0 1 1-e 0+e 0+e],c2);patch([0 0 1 1-e 0+e 0+e],[0 1 1 1-e 1-e 0+e],c3);plot([0 1 1 0 0],[0 0 1 1 0]);
  wires:
  poslin/1 -> "a{3}"/1
  netsum/1 -> poslin/1
  "b{3}"/1 -> netsum/2
  "LW{3,2}"/1("lz{3,2}") -> netsum/1
  Delays 1/1("pd{3,2}") -> "LW{3,2}"/1("ad{3,2}")
  "a{2} "/1 -> Delays 1/1("p{3}")

== system AcasXuClosedLoop_all_comp/networks/Networks_m_5/AcasXu_2_5/Layer 4 ==
  "a{3} " : Inport  PortDimensions=50
      mask Display: e = 0.06;c1 = [120 230 180]/255;c2 = ([120 230 180]+0)/(256+128);c3 = ([120 230 180]+128)/(256+128);patch([0 1 1 0],[0 0 1 1],c1);patch([0 1 1 1-e 1-e 0+e],[0 0 1 1-e 0+e 0+e],c2);patch([0 0 1 1-e 0+e 0+e],[0 1 1 1-e 1-e 0+e],c3);plot([0 1 1 0 0],[0 0 1 1 0]);
  Delays 1 : Subsystem
      mask Display: e = 0.06;c1 = [120 150 230]/255;c2 = ([120 150 230]+0)/383;c3 = ([120 150 230]+128)/383;patch([0 1 1 0],[0 0 1 1],c1);patch([0 1 1 1-e 1-e 0+e],[0 0 1 1-e 0+e 0+e],c2);patch([0 0 1 1-e 0+e 0+e],[0 1 1 1-e 1-e 0+e],c3);plot([0 1 1 0 0],[0 0 1 1 0]);disp('0');
  "LW{4,3}" : Subsystem
      mask Display: e = 0.06;c1 = [120 150 230]/255;c2 = ([120 150 230]+0)/383;c3 = ([120 150 230]+128)/383;patch([0 1 1 0],[0 0 1 1],c1);patch([0 1 1 1-e 1-e 0+e],[0 0 1 1-e 0+e 0+e],c2);patch([0 0 1 1-e 0+e 0+e],[0 1 1 1-e 1-e 0+e],c3);plot([0 1 1 0 0],[0 0 1 1 0]);disp('W');
  "b{4}" : Constant  Value=[1.548010000000000108144604382687248289585113525390625;-0.098168800000000000505195885125431232154369354248046875;-1.6671400000000000662936372464173473417758941650390625;0.118808999999999997942978779974509961903095245361328125;-0.3544169999999999820516904946998693048954010009765625;-0.43581599999999998118482835707254707813262939453125;-1.427259999999999973141484588268212974071502685546875;0.038447000000000002006839139312432962469756603240966796875;0.440151000000000014455991959039238281548023223876953125;0.921942000000000039250380723387934267520904541015625;-0.85051299999999996348520880928845144808292388916015625;0.1774010000000000031317171078626415692269802093505859375;-0.139890999999999987579712978913448750972747802734375;-2.13018999999999980587972459034062922000885009765625;-3.0294200000000000017053025658242404460906982421875;0.438805999999999973848474610349512659013271331787109375;-1.0520300000000000206767936106189154088497161865234375;-0.08207820000000000393303167811609455384314060211181640625;-0.6246080000000000520543608217849396169185638427734375;-1.57336000000000009180212146020494401454925537109375;-0.9218180000000000262616595136933028697967529296875;-1.063660000000000049880100050359033048152923583984375;-0.343635999999999997012167796128778718411922454833984375;-0.388892999999999988691712360378005541861057281494140625;-0.29755399999999998517097310468670912086963653564453125;-1.1142499999999999626965063725947402417659759521484375;-1.8420300000000000562039303986239247024059295654296875;-0.82963500000000001133315663537359796464443206787109375;1.033160000000000078301809480763040482997894287109375;-0.295208999999999999186428567554685287177562713623046875;-1.919610000000000038511416278197430074214935302734375;0.38875300000000001521271997262374497950077056884765625;-0.7366580000000000350013351635425351560115814208984375;-0.01055799999999999967681407753161693108268082141876220703125;-0.3388109999999999732978039901354350149631500244140625;1.644249999999999989341858963598497211933135986328125;-0.56623900000000004784084239872754551470279693603515625;-1.539320000000000021600499167107045650482177734375;0.1086580000000000045812242888132459484040737152099609375;0.1382440000000000057678306575326132588088512420654296875;-0.50385800000000002807354348988155834376811981201171875;-0.0817628999999999994674482195478049106895923614501953125;-0.09210690000000000543511902151294634677469730377197265625;1.1767600000000000282085466096759773790836334228515625;-0.206857000000000013084644479022244922816753387451171875;1.4793600000000000083133500083931721746921539306640625;-0.8695239999999999636060010743676684796810150146484375;0.494456000000000006622258297284133732318878173828125;0.8987439999999999873381284487550146877765655517578125;-0.088381199999999993099919493033667095005512237548828125]
      mask Display: e = 0.06;c1 = [120 150 230]/255;c2 = ([120 150 230]+0)/383;c3 = ([120 150 230]+128)/383;patch([0 1 1 0],[0 0 1 1],c1);patch([0 1 1 1-e 1-e 0+e],[0 0 1 1-e 0+e 0+e],c2);patch([0 0 1 1-e 0+e 0+e],[0 1 1 1-e 1-e 0+e],c3);plot([0 1 1 0 0],[0 0 1 1 0]);disp('b');
  netsum : Sum  Inputs=2  SaturateOnIntegerOverflow=on  InputSameDT=on  OutDataTypeStr=Inherit: Same as first input
      mask Display: e = 0.06;c1 = [120 150 230]/255;c2 = ([120 150 230]+0)/383;c3 = ([120 150 230]+128)/383;patch([0 1 1 0],[0 0 1 1],c1);patch([0 1 1 1-e 1-e 0+e],[0 0 1 1-e 0+e 0+e],c2);patch([0 0 1 1-e 0+e 0+e],[0 1 1 1-e 1-e 0+e],c3);plot([0 1 1 0 0],[0 0 1 1 0]);disp('+');
  poslin : neural/Transfer Functions/poslin
      settings: SourceProductName=Deep Learning Toolbox  SourceProductBaseCode=NN
  "a{4}" : Outport  InitialOutput=[0;0;0;0;0;0;0;0;0;0;0;0;0;0;0;0;0;0;0;0;0;0;0;0;0;0;0;0;0;0;0;0;0;0;0;0;0;0;0;0;0;0;0;0;0;0;0;0;0;0]
      mask Display: e = 0.06;c1 = [120 230 180]/255;c2 = ([120 230 180]+0)/(256+128);c3 = ([120 230 180]+128)/(256+128);patch([0 1 1 0],[0 0 1 1],c1);patch([0 1 1 1-e 1-e 0+e],[0 0 1 1-e 0+e 0+e],c2);patch([0 0 1 1-e 0+e 0+e],[0 1 1 1-e 1-e 0+e],c3);plot([0 1 1 0 0],[0 0 1 1 0]);
  wires:
  poslin/1 -> "a{4}"/1
  netsum/1 -> poslin/1
  "b{4}"/1 -> netsum/2
  "LW{4,3}"/1("lz{4,3}") -> netsum/1
  Delays 1/1("pd{4,3}") -> "LW{4,3}"/1("ad{4,3}")
  "a{3} "/1 -> Delays 1/1("p{4}")
